simulink model "TosAeb"  (R2021a)
settings: Solver=FixedStepDiscrete  StartTime=0.0  StopTime=inf  FixedStep=0.01  RelTol=1e-3
undefined symbols (not defined in the file or in its folder): ACCModSts, AccSts1, AEB_ObjectMovingOrientation, AEB_ObjectMovingState, CN_BTCActive, CN_CurrentGearR, CN_CurrentGearR_DHT, CN_PTCActive, CN_VDCActive, CN_VSC_EspOff, CN_VSC_FCW_AEB_Error, CN_VSC_FCW_AEB_NoDispay, CN_VSC_FCW_AEB_PerfmDgdtn, CN_VSC_FCW_AEB_TempUnAvlbl, CN_VSC_VehStandstill, CNaccelAvoidMax, CNaccelAvoidMin, CNbrakePedalPress, CNcomfortStrLeftAccelMax, CNcomfortStrLeftAccelMin, CNcomfortStrRightAccelMax, CNcomfortStrRightAccelMin, CNDrvDoorOpen, CNDrvModeEco, CNDrvModeNorm, CNDrvModeOther, CNDrvModeSport, CNDrvSBRreminder, CNNull, CNobjInvalidID, CNPassDoorOpen, CNPassSBRreminder, CNPEBSAccelNegInf, CNPEBSAccelNull, CNPEBSTimeInf, CNPEBSTimeNull, CNSTOPMAX, CNSTOPNULL, CNsubjWidth, CNSwtOFF, CNSwtON, CNTTCMAX, CNTTCNULL, CNVehConfigAEBCycNone, CNVehConfigAEBNone, CNVehConfigAEBPedNone, CNVehConfigFCWNone, CNVehConfigJANone, CollisionSeverity, FALSE, FCWSnvty, JACycObjCalcInfoDefaultParams, JAPedObjCalcInfoDefaultParams, JAVehObjCalcInfoDefaultParams, latCycObjCalcInfoDefaultParams, latPedObjCalcInfoDefaultParams, longObjCalcInfoDefaultParams, ModSts, movingStatus, ObjectType ... (+1112)
libraries: simulink x3031, AEBSFunctionLib x50, Ta_Library x33, AEBSStateMachineLib x23, Mylibrary x12, simulink_extras x7, ObjSelect_lib x3, dspstat3 x2
(part 36 of 102)

== system TosAeb/AEB_Objsel/TA_InfoRebuild/TAObjInfoCalc/For Each Subsystem/Info_rebuild/Road_relative/LineInfo_Process/LineInfo_Process_Right ==
  Rightline_Data : Inport
  Bus Creator : BusCreator  Inputs=7
  Bus Selector : BusSelector  OutputSignals=lineSegNum,lineSegs
  Bus Selector1 : BusSelector  OutputSignals=lineSegValid,lineSegStart,lineSegEnd,lineC0,lineC1,lineC2,lineC3
  Bus Selector2 : BusSelector  OutputSignals=lineSegValid,lineSegStart,lineSegEnd,lineC0,lineC1,lineC2,lineC3
  Bus Selector3 : BusSelector  OutputSignals=lineSegValid,lineSegStart,lineSegEnd,lineC0,lineC1,lineC2,lineC3
  Data Type Conversion : DataTypeConversion  OutDataTypeStr=boolean
  Data Type Conversion1 : DataTypeConversion  OutDataTypeStr=boolean
  Data Type Conversion2 : DataTypeConversion  OutDataTypeStr=boolean
  Line_Info : MATLAB Function  TreatAsAtomicUnit=on
  Selector18 : Selector  IndexMode=Zero-based  InputPortWidth=3  IndexOptions=Index vector (dialog)  Indices=[0]  OutputSizes=1
  Selector2 : Selector  IndexMode=Zero-based  InputPortWidth=3  IndexOptions=Index vector (dialog)  Indices=[1]  OutputSizes=1
  Selector3 : Selector  IndexMode=Zero-based  InputPortWidth=3  IndexOptions=Index vector (dialog)  Indices=[2]  OutputSizes=1
  RightLine_Info : Outport
  wires:
  Rightline_Data/1 -> Bus Selector/1
  Selector3/1 -> Bus Selector3/1
  Selector18/1 -> Bus Selector1/1
  Selector2/1 -> Bus Selector2/1
  Bus Selector/2 -> Selector3/1, Selector2/1, Selector18/1  "<lineSegs>"
  Bus Selector1/1 -> Data Type Conversion/1  "<lineSegValid>"
  Bus Selector1/2 -> Line_Info/3(seg1_Start)  "<lineSegStart>"
  Bus Selector1/3 -> Line_Info/4(seg1_end)  "<lineSegEnd>"
  Bus Selector1/4 -> Line_Info/5(seg1_C0)  "<lineC0>"
  Bus Selector1/5 -> Line_Info/6(seg1_C1)  "<lineC1>"
  Bus Selector1/6 -> Line_Info/7(seg1_C2)  "<lineC2>"
  Bus Selector1/7 -> Line_Info/8(seg1_C3)  "<lineC3>"
  Bus Selector2/1 -> Data Type Conversion1/1  "<lineSegValid>"
  Bus Selector2/2 -> Line_Info/10(seg2_Start)  "<lineSegStart>"
  Bus Selector2/3 -> Line_Info/11(seg2_end)  "<lineSegEnd>"
  Bus Selector2/4 -> Line_Info/12(seg2_C0)  "<lineC0>"
  Bus Selector2/5 -> Line_Info/13(seg2_C1)  "<lineC1>"
  Bus Selector2/6 -> Line_Info/14(seg2_C2)  "<lineC2>"
  Bus Selector2/7 -> Line_Info/15(seg2_C3)  "<lineC3>"
  Bus Selector3/1 -> Data Type Conversion2/1  "<lineSegValid>"
  Bus Selector3/2 -> Line_Info/17(seg3_Start)  "<lineSegStart>"
  Bus Selector3/3 -> Line_Info/18(seg3_end)  "<lineSegEnd>"
  Bus Selector3/4 -> Line_Info/19(seg3_C0)  "<lineC0>"
  Bus Selector3/5 -> Line_Info/20(seg3_C1)  "<lineC1>"
  Bus Selector3/6 -> Line_Info/21(seg3_C2)  "<lineC2>"
  Bus Selector3/7 -> Line_Info/22(seg3_C3)  "<lineC3>"
  Bus Selector/1 -> Line_Info/1(seg_num)  "<lineSegNum>"
  Line_Info/1(Valid) -> Bus Creator/1
  Line_Info/2(Line_start) -> Bus Creator/2
  Line_Info/3(Line_end) -> Bus Creator/3
  Line_Info/4(C0) -> Bus Creator/4
  Line_Info/5(C1) -> Bus Creator/5
  Line_Info/6(C2) -> Bus Creator/6
  Line_Info/7(C3) -> Bus Creator/7
  Bus Creator/1 -> RightLine_Info/1
  Data Type Conversion/1 -> Line_Info/2(seg1_valid)
  Data Type Conversion1/1 -> Line_Info/9(seg2_valid)
  Data Type Conversion2/1 -> Line_Info/16(seg3_valid)

== system TosAeb/AEB_Objsel/TA_InfoRebuild/TAObjInfoCalc/For Each Subsystem/Info_rebuild/Road_relative/RebuildInfo_Process/If Action Subsystem ==
  Action Port : ActionPort  ActionPortLabel=if(u1 > 0)
  Constant : Constant  Value=0  OutDataTypeStr=single
  Constant1 : Constant  Value=0  OutDataTypeStr=single
  Constant2 : Constant  Value=0  OutDataTypeStr=uint8
  objLatDist2LaneCenter : Outport
  objLatDist2EgoCenter : Outport  Port=2
  objPosition : Outport  Port=3
  wires:
  Constant2/1 -> objPosition/1
  Constant1/1 -> objLatDist2EgoCenter/1
  Constant/1 -> objLatDist2LaneCenter/1

== system TosAeb/AEB_Objsel/TA_InfoRebuild/TAObjInfoCalc/For Each Subsystem/Info_rebuild/Road_relative/RebuildInfo_Process/If Action Subsystem1 ==
  Obj_Infomation : Inport
  LeftLine_Info : Inport  Port=2
  RightLine_Info : Inport  Port=3
  Action Port : ActionPort  ActionPortLabel=else
  Bus Selector : BusSelector  OutputSignals=PosX_s,PosY_s
  Bus Selector1 : BusSelector  OutputSignals=Valid,C0,C1,C2,C3
  Bus Selector2 : BusSelector  OutputSignals=Valid,C0,C1,C2,C3
  MATLAB Function : MATLAB Function  TreatAsAtomicUnit=on
  objLatDist2LaneCenter : Outport
  objLatDist2EgoCenter : Outport  Port=2
  objPosition : Outport  Port=3
  wires:
  Obj_Infomation/1 -> Bus Selector/1
  LeftLine_Info/1 -> Bus Selector1/1
  RightLine_Info/1 -> Bus Selector2/1
  Bus Selector/1 -> MATLAB Function/1(dx)  "<PosX_s>"
  Bus Selector/2 -> MATLAB Function/2(dy)  "<PosY_s>"
  Bus Selector1/2 -> MATLAB Function/4(left_C0)  "<C0>"
  Bus Selector1/1 -> MATLAB Function/3(left_valid)  "<Valid>"
  Bus Selector1/3 -> MATLAB Function/5(left_C1)  "<C1>"
  Bus Selector1/4 -> MATLAB Function/6(left_C2)  "<C2>"
  Bus Selector1/5 -> MATLAB Function/7(left_C3)  "<C3>"
  Bus Selector2/1 -> MATLAB Function/8(right_valid)  "<Valid>"
  Bus Selector2/2 -> MATLAB Function/9(right_C0)  "<C0>"
  Bus Selector2/3 -> MATLAB Function/10(right_C1)  "<C1>"
  Bus Selector2/4 -> MATLAB Function/11(right_C2)  "<C2>"
  Bus Selector2/5 -> MATLAB Function/12(right_C3)  "<C3>"
  MATLAB Function/1(objLatDist2LaneCenter) -> objLatDist2LaneCenter/1
  MATLAB Function/2(objLatDist2EgoCenter) -> objLatDist2EgoCenter/1
  MATLAB Function/3(objPosition) -> objPosition/1

== system TosAeb/AEB_Objsel/TA_InfoRebuild/TAObjInfoCalc/For Each Subsystem/Info_rebuild/Road_relative/RebuildInfo_Process/Subsystem ==
  Obj_Infomation : Inport
  Bus Selector : BusSelector  OutputSignals=ID,Valid
  Compare To Constant : simulink/Logic and Bit Operations/Compare To Constant  relop===  const=0  OutDataTypeStr=boolean
  Compare To Constant1 : simulink/Logic and Bit Operations/Compare To Constant  relop===  const=0  OutDataTypeStr=boolean
  Data Type Conversion : DataTypeConversion  OutDataTypeStr=uint8
  Logical Operator : Logic  Operator=OR  AllPortsSameDT=off  OutDataTypeStr=boolean
  if_judge : Outport
  wires:
  Obj_Infomation/1 -> Bus Selector/1
  Bus Selector/1 -> Compare To Constant/1  "<ID>"
  Bus Selector/2 -> Compare To Constant1/1  "<Valid>"
  Compare To Constant/1 -> Logical Operator/1
  Compare To Constant1/1 -> Logical Operator/2
  Logical Operator/1 -> Data Type Conversion/1
  Data Type Conversion/1 -> if_judge/1

== system TosAeb/AEB_Objsel/TA_Processor/AEB_ObjSelect/TargetInfoCheck/Data_precess/TA_EffectCalculate/Obj_FilterFlgCalculate/JA_ObjFilter ==
  TA_ObjInfo : Inport
  PahEstimn : Inport  Port=2
  VehSelfData : Inport  Port=3
  Bus Selector : BusSelector  OutputSignals=VehSelf.ABS_VehicleSpeed,VehSelf.AX1,VehSelf.AY1,VehSelf.YawRate
  Bus Selector1 : BusSelector  OutputSignals=TA_ObsCenterAngle,TA_ObsOrientation,TA_ObsLength,TA_ObsWidth,TA_ObsDx,TA_ObsDy,TA_ObsAy,TA_ObsID,TA_ObsVy,TA_ObsVxAbs,TA_ObsAxAbs
  CollisionProbCalc : Subsystem
  CollisionProbCalcFlow : Stateflow chart  TreatAsAtomicUnit=on
  Constant1 : Constant  Value=100  OutDataTypeStr=single
  Constant2 : Constant  Value=0.02  OutDataTypeStr=single
  Constant3 : Constant  Value=2  OutDataTypeStr=single
  JAObjEnable : Subsystem
  Logical Operator : Logic  Operator=NOT  AllPortsSameDT=off  OutDataTypeStr=boolean
  Switch : Switch  Criteria=u2 ~= 0
  ObjColliInfo : Outport
  wires:
  VehSelfData/1 -> Bus Selector/1
  Bus Selector/2 -> CollisionProbCalc/3(subjLongAccel)  "<AX1>"
  [CrossFlg] -> CollisionProbCalc/Trigger
  PahEstimn/1 -> CollisionProbCalc/17(PahEstimn)
  CollisionProbCalc/1(ObjColliFlg) -> CollisionProbCalcFlow/3(preCollisionProb)  "ObjColliFlg"
  CollisionProbCalcFlow/1(TTC) -> Switch/3  "tPredictTime"
  Bus Selector1/2 -> CollisionProbCalc/7(ObjectMovingOrientation)  "<TA_ObsOrientation>"
  Constant3/1 -> CollisionProbCalcFlow/2(P_JaPedCA_PreCollisionPredTimeMax)
  CollisionProbCalcFlow/3(CollisionProbFunc()) -> [CrossFlg]
  Bus Selector/3 -> CollisionProbCalc/4(subjLatAccel)  "<AY1>"
  Bus Selector/4 -> CollisionProbCalc/5(subjYawRate)  "<YawRate>"
  CollisionProbCalcFlow/2(tPredictTimeOut) -> CollisionProbCalc/1(tPredTime)
  Bus Selector1/1 -> CollisionProbCalc/6(ObjectYawAngle)  "<TA_ObsCenterAngle>"
  Constant2/1 -> CollisionProbCalcFlow/1(P_JaPedCA_PreCollisionStepTime)
  Bus Selector1/3 -> CollisionProbCalc/8(ObjectLength)  "<TA_ObsLength>"
  Bus Selector/1 -> CollisionProbCalc/2(subjVel)  "<ABS_VehicleSpeed>"
  Bus Selector1/4 -> CollisionProbCalc/9(ObjectWidth)  "<TA_ObsWidth>"
  Bus Selector1/5 -> CollisionProbCalc/10(dx)  "<TA_ObsDx>"
  Bus Selector1/6 -> CollisionProbCalc/11(dy)  "<TA_ObsDy>"
  Bus Selector1/7 -> CollisionProbCalc/12(ay)  "<TA_ObsAy>"
  Bus Selector1/8 -> CollisionProbCalc/13(ObjectID)  "<TA_ObsID>"
  Bus Selector1/9 -> CollisionProbCalc/14(vy)  "<TA_ObsVy>"
  Bus Selector1/10 -> CollisionProbCalc/15(vxAbsObj)  "<TA_ObsVxAbs>"
  Bus Selector1/11 -> CollisionProbCalc/16(axAbsObj)  "<TA_ObsAxAbs>"
  TA_ObjInfo/1 -> JAObjEnable/1(TA_ObjInfo), [TA_ObjInfo]
  [TA_ObjInfo] -> Bus Selector1/1
  JAObjEnable/1(JAObjEnable) -> Logical Operator/1
  Logical Operator/1 -> Switch/2
  Switch/1 -> ObjColliInfo/1
  Constant1/1 -> Switch/1  "ObjColliFlg"

== system TosAeb/AEB_Objsel/TA_Processor/AEB_ObjSelect/TargetInfoCheck/Data_precess/TA_EffectCalculate/Obj_FilterFlgCalculate/Lat_ObjFilter ==
  Utility : Inport
  TA_ObjInfo : Inport  Port=2
  VehSelfData : Inport  Port=3
  If : If
  Lat_Objelse : Subsystem  TreatAsAtomicUnit=on
  Lat_Objif : Subsystem  TreatAsAtomicUnit=on
  Merge : Merge
  Merge1 : Merge
  Merge2 : Merge
  Subsystem : Subsystem
  LatObj_Valid : Outport
  Lat_ObjIntersect : Outport  Port=2
  Lat_TTR : Outport  Port=3
  wires:
  Subsystem/1(Obj_IDFlg) -> If/1
  Utility/1 -> Subsystem/1(TA_ObjID)
  If/1 -> Lat_Objif/Action
  If/2 -> Lat_Objelse/Action
  VehSelfData/1 -> Lat_Objelse/2(VehSelfData)
  TA_ObjInfo/1 -> Lat_Objelse/1(TA_ObjInfo)
  Lat_Objif/1(LatObj_Valid) -> Merge/1
  Lat_Objelse/1(LatObj_Valid) -> Merge/2
  Merge/1 -> LatObj_Valid/1
  Lat_Objif/2(Lat_ObjIntersect) -> Merge1/1
  Lat_Objelse/2(Lat_ObjIntersect) -> Merge1/2
  Lat_Objif/3(Lat_TTR) -> Merge2/1
  Lat_Objelse/3(Lat_TTR) -> Merge2/2
  Merge1/1 -> Lat_ObjIntersect/1
  Merge2/1 -> Lat_TTR/1

== system TosAeb/AEB_Objsel/TA_Processor/AEB_ObjSelect/TargetInfoCheck/Data_precess/TA_EffectCalculate/Obj_FilterFlgCalculate/Long_ObjFilter ==
  Obj_WeightCoef : Inport
  TA_ObjInfo : Inport  Port=2
  VehSelfData : Inport  Port=3
  " PahEstimn" : Inport  Port=4
  Info_Calculate : Subsystem
  OverlapCalculate : Subsystem
  TTR_Calculate : Subsystem
  Boudingbox_Calculate : Outport
  Obj_Coef : Outport  Port=2
  TTI : Outport  Port=3
  Long_ObjWeightCoef : Outport  Port=4
  wires:
  Obj_WeightCoef/1 -> Info_Calculate/1(Obj_WeightCoef)
  TA_ObjInfo/1 -> Info_Calculate/2(TA_ObjInfo), [TA_ObjInfo]
  VehSelfData/1 -> [VehSelfData], Info_Calculate/3(VehSelfData)
  Info_Calculate/1(Long_ObjWeightCoef) -> Long_ObjWeightCoef/1, TTR_Calculate/1(Long_ObjWeightCoef)
  TTR_Calculate/2(TimeToReachLgt) -> [TTI], OverlapCalculate/2(TimeToReachLgt)
  TTR_Calculate/1(Long_ObjWeightCoefTTI) -> OverlapCalculate/1(Long_ObjWeightCoefTTI), [Obj_Coef]
  [TA_ObjInfo] -> TTR_Calculate/2(TA_ObjInfo)
  [TA_ObjInfo] -> OverlapCalculate/4(TA_ObjInfo)
  [VehSelfData] -> TTR_Calculate/3(VehSelfData)
  [VehSelfData] -> OverlapCalculate/5(VehSelfData)
  " PahEstimn"/1 -> OverlapCalculate/3(PahEstimn)
  OverlapCalculate/1(Boudingbox_Calculate) -> Boudingbox_Calculate/1
  [Obj_Coef] -> Obj_Coef/1
  [TTI] -> TTI/1

== system TosAeb/AEB_Objsel/TA_Processor/AEB_ObjSelect/TargetInfoCheck/Data_precess/TA_EffectCalculate/Obj_FilterFlgCalculate/WeightCoef_Cal ==
  note: 是否要根据有效目标降低循环维度，以减少for循环运算次数
  Overlap_Current : Inport
  Utility : Inport  Port=2
  Overlap_Prediction : Inport  Port=3
  VehSelfData : Inport  Port=4
  Bus Selector : BusSelector  OutputSignals=VehSelf.AEB_Intervention_ESP
  Compare To Constant : simulink/Logic and Bit Operations/Compare To Constant  relop===  const=0  OutDataTypeStr=boolean
  Compare To Constant{1,4..7} (5 alike) : simulink/Logic and Bit Operations/Compare To Constant  relop===  const=1  OutDataTypeStr=boolean
  Compare To Constant2 : simulink/Logic and Bit Operations/Compare To Constant  relop===  const=0  OutDataTypeStr=boolean
  Compare To Constant3 : simulink/Logic and Bit Operations/Compare To Constant  relop===  const=0  OutDataTypeStr=boolean
  Constant : Constant  Value=255  OutDataTypeStr=uint8
  Constant1 : Constant  OutDataTypeStr=uint8
  Constant2 : Constant  Value=2  OutDataTypeStr=uint8
  Constant3 : Constant  Value=3  OutDataTypeStr=uint8
  Constant4 : Constant  Value=0  OutDataTypeStr=uint8
  Logical Operator : Logic  AllPortsSameDT=off  OutDataTypeStr=boolean
  Logical Operator1 : Logic  AllPortsSameDT=off  OutDataTypeStr=boolean
  Logical Operator2 : Logic  AllPortsSameDT=off  OutDataTypeStr=boolean
  Switch{,1..4} (5 alike) : Switch  Criteria=u2 ~= 0
  WeightCoef_Cal1 : MATLAB Function  Commented=on  TreatAsAtomicUnit=on
  Obj_WeightCoef : Outport
  wires:
  Utility/1 -> Compare To Constant/1
  Compare To Constant/1 -> Switch/2
  Constant/1 -> Switch/1
  Overlap_Current/1 -> Compare To Constant5/1, Compare To Constant3/1, Compare To Constant1/1
  Overlap_Prediction/1 -> Compare To Constant6/1, Compare To Constant4/1, Compare To Constant2/1
  Compare To Constant1/1 -> Switch1/1, Logical Operator/1
  Compare To Constant2/1 -> Logical Operator/2
  Logical Operator/1 -> Switch2/2
  Constant1/1 -> Switch2/1
  Logical Operator1/1 -> Switch3/2
  Compare To Constant3/1 -> Logical Operator1/1
  Compare To Constant4/1 -> Logical Operator1/2
  Constant2/1 -> Switch3/1
  Compare To Constant5/1 -> Logical Operator2/1
  Compare To Constant6/1 -> Logical Operator2/2
  Logical Operator2/1 -> Switch4/2
  Constant3/1 -> Switch4/1
  Switch4/1 -> Switch3/3
  Switch3/1 -> Switch2/3
  Switch2/1 -> Switch1/3
  Constant4/1 -> Switch4/3
  Switch/1 -> Obj_WeightCoef/1
  VehSelfData/1 -> Bus Selector/1
  Compare To Constant7/1 -> Switch1/2
  Switch1/1 -> Switch/3
  Bus Selector/1 -> Compare To Constant7/1  "<AEB_Intervention_ESP>"

== system TosAeb/AEB_Objsel/TA_Processor/AEB_ObjSelect/TargetInfoCheck/Data_precess/TA_EffectCalculate/ValideateObj/ObjPos_Calculate ==
  TA_ObsInfo : Inport
  Obj_Enable : Inport  Port=2
  PathEstimn : Inport  Port=3
  If : If
  Merge : Merge
  Merge1 : Merge
  ObjPos_Calculateelse : Subsystem  TreatAsAtomicUnit=on
  ObjPos_Calculateif : Subsystem  TreatAsAtomicUnit=on
  Subsystem : Subsystem
  Utility : Outport
  Overlap_Current : Outport  Port=2
  Overlap_Prediction : Outport  Port=3
  wires:
  Obj_Enable/1 -> Subsystem/1(Obj_Enable)
  Subsystem/2(Obj_Flg) -> If/1
  If/1 -> ObjPos_Calculateif/Action
  If/2 -> ObjPos_Calculateelse/Action
  TA_ObsInfo/1 -> ObjPos_Calculateelse/1(TA_ObsInfo)
  PathEstimn/1 -> ObjPos_Calculateelse/2(PathEstimn)
  Subsystem/1(Utility) -> Utility/1
  ObjPos_Calculateif/1(Overlap_Current) -> Merge/1
  ObjPos_Calculateelse/1(Overlap_Current) -> Merge/2
  ObjPos_Calculateif/2(Overlap_Prediction) -> Merge1/1
  ObjPos_Calculateelse/2(Overlap_Prediction) -> Merge1/2
  Merge/1 -> Overlap_Current/1
  Merge1/1 -> Overlap_Prediction/1

== system TosAeb/AEB_Objsel/TA_Processor/AEB_ObjSelect/TargetInfoCheck/Data_precess/TA_EffectCalculate/ValideateObj/ValidObj_PreProcess ==
  TA_ObjInfo : Inport
  VehSelfData : Inport  Port=2
  Bus Selector : BusSelector  OutputSignals=TA_ObsType,TA_ObsOrientation,TA_ObsDx,TA_ObsDy,TA_ObsVx,TA_ObsConfidence,TA_ObsVxAbs
  Bus Selector1 : BusSelector  OutputSignals=TA_ObsID
  Bus Selector2 : BusSelector  OutputSignals=VehSelf.ABS_VehicleSpeed
  If : If
  If Action Subsystem : Subsystem  TreatAsAtomicUnit=on
  If Action Subsystem1 : Subsystem  TreatAsAtomicUnit=on
  Merge : Merge
  Subsystem : Subsystem
  Obj_Enable : Outport
  wires:
  Subsystem/1(Obj_IDFlg) -> If/1
  If/1 -> If Action Subsystem/Action
  If/2 -> If Action Subsystem1/Action
  If Action Subsystem/1(Obj_Enable) -> Merge/1
  If Action Subsystem1/1(Obj_Enable) -> Merge/2
  Merge/1 -> Obj_Enable/1
  TA_ObjInfo/1 -> Bus Selector/1, Bus Selector1/1
  VehSelfData/1 -> Bus Selector2/1
  Bus Selector2/1 -> If Action Subsystem1/8(Veh_LongSpd_mps)  "<ABS_VehicleSpeed>"
  Bus Selector1/1 -> Subsystem/1(TA_ObsID)  "<TA_ObsID>"
  Bus Selector/1 -> If Action Subsystem1/1(TA_ObsType)  "<TA_ObsType>"
  Bus Selector/2 -> If Action Subsystem1/2(TA_ObjBehavior)  "<TA_ObsOrientation>"
  Bus Selector/3 -> If Action Subsystem1/3(TA_Obsdx)  "<TA_ObsDx>"
  Bus Selector/4 -> If Action Subsystem1/4(TA_Obsdy)  "<TA_ObsDy>"
  Bus Selector/5 -> If Action Subsystem1/5(TA_Obsvx)  "<TA_ObsVx>"
  Bus Selector/6 -> If Action Subsystem1/6(TA_ObsQuality)  "<TA_ObsConfidence>"
  Bus Selector/7 -> If Action Subsystem1/7(TA_ObsVxAbs)  "<TA_ObsVxAbs>"

== system TosAeb/AEB_Objsel/TA_Processor/PreProcessor/ObjectPrecessor/TAObjInfoCalc/For Each Subsystem/ForEachInput/PosErrorCalc ==
  Pos_x : Inport
  ObsID_u8 : Inport  Port=2
  Add : Sum
  Add1 : Sum  Inputs=+-
  Compare To Constant3 : simulink/Logic and Bit Operations/Compare To Constant  relop=<  const=0  OutDataTypeStr=boolean
  Constant : Constant  Value=262  OutDataTypeStr=single
  Constant1 : Constant  Value=P_TA_PrmForVehRearAxleDisttoFrnt  OutDataTypeStr=single
  FakeObjJudge : Stateflow chart  TreatAsAtomicUnit=on
  Logical Operator : Logic  Operator=NOT  AllPortsSameDT=off  OutDataTypeStr=boolean
  Max : MinMax  Function=max  Inputs=2
  Switch : Switch  Criteria=u2 ~= 0
  Unary Minus : UnaryMinus
  Unit Delay2 : UnitDelay  SampleTime=-1  HasFrameUpgradeWarning=on
  ObsPosX_s : Outport
  wires:
  ObsID_u8/1 -> Unit Delay2/1, FakeObjJudge/1(ID)
  Unit Delay2/1 -> FakeObjJudge/2(LastID)
  Pos_x/1 -> [Pos_x], Switch/3, Compare To Constant3/1
  Compare To Constant3/1 -> FakeObjJudge/3(FakeFlg)
  FakeObjJudge/1(ConfFlg) -> Logical Operator/1
  Logical Operator/1 -> Switch/2
  [Pos_x] -> Unary Minus/1
  Unary Minus/1 -> Add/1
  Add/1 -> Switch/1
  Constant/1 -> Add/2
  Constant1/1 -> Add1/2
  Switch/1 -> Max/1
  Max/1 -> Add1/1
  [Pos_x] -> Max/2
  Add1/1 -> ObsPosX_s/1

== system TosAeb/AEB_Objsel/TA_Processor/PreProcessor/ObjectPrecessor/TAObjInfoCalc/For Each Subsystem/Info_Check1/BasicInfo_check ==
  ID_change : Inport
  Fus_ObjState : Inport  Port=2
  Fus_Type : Inport  Port=3
  ObsID_u8 : Inport  Port=4
  ObsConfidence_u8 : Inport  Port=5
  ObsPosX_s : Inport  Port=6
  ObsPosY_s : Inport  Port=7
  ObsVelX_s : Inport  Port=8
  ObsVelY_s : Inport  Port=9
  ObsAccX_s : Inport  Port=10
  ObsPosXSTD_s : Inport  Port=11
  ObsPosYSTD_s : Inport  Port=12
  ObsVelXSTD_s : Inport  Port=13
  ObsVelYSTD_s : Inport  Port=14
  ObsAccY_s : Inport  Port=15
  ObsVelXAbs_s : Inport  Port=16
  ObsVelYAbs_s : Inport  Port=17
  ObsAngle_s : Inport  Port=18
  ObsAngleSTD_s : Inport  Port=19
  Veh_AEBState : Inport  Port=20
  Vhe_Spd : Inport  Port=21
  Veh_YawRate : Inport  Port=22
  Veh_DrvAcc : Inport  Port=23
  Veh_DrvAy : Inport  Port=24
  AbsSpd : Subsystem
  AccCorrect : Subsystem
  DxCorrect : Subsystem
  DyCorrect : Subsystem
  HeadAngle : Subsystem
  IDProcess : Subsystem
  QuaCorrect : Subsystem
  VxCorrect : Subsystem
  VyCorrect : Subsystem
  Fus_ObjDy : Outport
  Fus_ObjDx : Outport  Port=2
  Fus_ObjVx : Outport  Port=3
  Fus_ObjVy : Outport  Port=4
  Fus_ObjQuality : Outport  Port=5
  Fus_ObjAy : Outport  Port=6
  Fus_ObjAx : Outport  Port=7
  Fus_ObjVxAbs : Outport  Port=8
  Fus_ObjVyAbs : Outport  Port=9
  Fus_ObjHeadAngle : Outport  Port=10
  wires:
  [Obj_VxCr] -> AccCorrect/6(Obj_Vx)
  [Obj_Vy] -> DyCorrect/5(Fus_ObjVy_Single)
  ObsVelXSTD_s/1 -> VxCorrect/3(Fus_ObjVxStd)
  DxCorrect/2(Dx_CorrectFlg) -> [dx_Flg]
  ObsPosYSTD_s/1 -> DyCorrect/3(Fus_ObsDyStd)
  [Obj_ID] -> DxCorrect/1(Obj_ID)
  [dy_Flg] -> QuaCorrect/4(dy_flg)
  [Veh_AEBSta] -> DxCorrect/5(Veh_AEBFunState)
  DxCorrect/1(Fus_ObjDx) -> Fus_ObjDx/1
  [Obj_Dx] -> VxCorrect/4(Fus_ObjDx_Single)
  [Veh_AEBSta] -> VyCorrect/7(Veh_AEBFunState)
  ObsVelYSTD_s/1 -> VyCorrect/5(Fus_ObsVyStd)
  [Obj_Vx] -> DxCorrect/4(Fus_ObjVx_Single)
  ObsConfidence_u8/1 -> QuaCorrect/2(ObsConfidence_u8)
  VxCorrect/1(Fus_ObjVx) -> Fus_ObjVx/1, [Obj_VxCr]
  VxCorrect/2(Vx_CorrectFlg) -> [vx_Flg]
  [vy_Flg] -> QuaCorrect/7(vy_flg)
  ObsPosXSTD_s/1 -> DxCorrect/3(Fus_ObjDxQua_Single)
  [dx_Flg] -> QuaCorrect/5(dx_flg)
  [vx_Flg] -> QuaCorrect/6(vx_flg)
  QuaCorrect/1(Fus_ObjQuality) -> Fus_ObjQuality/1
  DyCorrect/2(Dy_CorrectFlg) -> [dy_Flg]
  VyCorrect/2(Vy_CorrectFlg) -> [vy_Flg]
  DyCorrect/1(Fus_ObjDy) -> Fus_ObjDy/1
  [Obj_ID] -> QuaCorrect/1(Obj_ID)
  AccCorrect/1(Fus_ObjAy) -> Fus_ObjAy/1
  Veh_AEBState/1 -> [Veh_AEBSta], DyCorrect/4(Veh_AEBFunState)
  [Veh_AEBSta] -> VxCorrect/5(Veh_AEBFunState)
  [Obj_ID] -> VyCorrect/1(Obj_ID)
  AccCorrect/2(Fus_ObjAx) -> Fus_ObjAx/1
  [Obj_ID] -> VxCorrect/1(Obj_ID)
  ObsPosX_s/1 -> [Obj_Dx], DxCorrect/2(Fus_ObjDx_Single)
  ObsVelX_s/1 -> VxCorrect/2(Fus_ObjVx_Single), [Obj_Vx]
  Fus_ObjState/1 -> VyCorrect/3(Fus_TargetState)
  ObsVelY_s/1 -> [Obj_Vy], VyCorrect/2(Fus_ObjVy_Single)
  [Obj_VyCr] -> AccCorrect/5(Obj_vy)
  VyCorrect/1(Fus_ObjVy) -> [Obj_VyCr], Fus_ObjVy/1
  [Veh_AEBSta] -> AccCorrect/7(Veh_AEBSta)
  ObsPosY_s/1 -> DyCorrect/2(Fus_ObjDy_Single), [Obj_Dy]
  [Obj_Dy] -> VyCorrect/6(Fus_ObjDy_Single)
  ID_change/1 -> IDProcess/1(ID_change)
  ObsID_u8/1 -> IDProcess/2(ObsID_u8)
  IDProcess/1(Obs_ID) -> [Obj_ID], DyCorrect/1(Obj_ID)
  Veh_YawRate/1 -> [Veh_YawRate], VyCorrect/4(Veh_YawRate)
  ObsAccX_s/1 -> AccCorrect/1(ObsAccX_s)
  ObsAccY_s/1 -> AccCorrect/2(ObsAccY_s)
  Veh_DrvAcc/1 -> AccCorrect/3(Veh_AccX)
  Veh_DrvAy/1 -> AccCorrect/4(Veh_AccY)
  [Obj_VyCr] -> AbsSpd/6(Obj_VyCr)
  [Obj_VxCr] -> AbsSpd/5(Obj_VxCr)
  [Veh_YawRate] -> AbsSpd/4(Veh_YawRate)
  Vhe_Spd/1 -> AbsSpd/3(Vhe_Spd)
  ObsVelXAbs_s/1 -> AbsSpd/1(ObsVelXAbs_s)
  ObsVelYAbs_s/1 -> AbsSpd/2(ObsVelYAbs_s)
  [Veh_AEBSta] -> QuaCorrect/3(Veh_AEBFunState)
  AbsSpd/1(Fus_ObjVxAbs) -> Fus_ObjVxAbs/1
  AbsSpd/2(Fus_ObjVyAbs) -> Fus_ObjVyAbs/1
  Fus_Type/1 -> HeadAngle/2(Fus_Type)
  ObsAngle_s/1 -> HeadAngle/3(ObsAngle_s)
  ObsAngleSTD_s/1 -> HeadAngle/4(ObsAngleSTD_s)
  [Obj_VxCr] -> HeadAngle/5(Obj_VxCr)
  [Obj_VyCr] -> HeadAngle/6(Obj_VyCr)
  [Obj_ID] -> HeadAngle/1(Obj_ID)
  HeadAngle/1(Fus_ObjHeadAngle) -> Fus_ObjHeadAngle/1

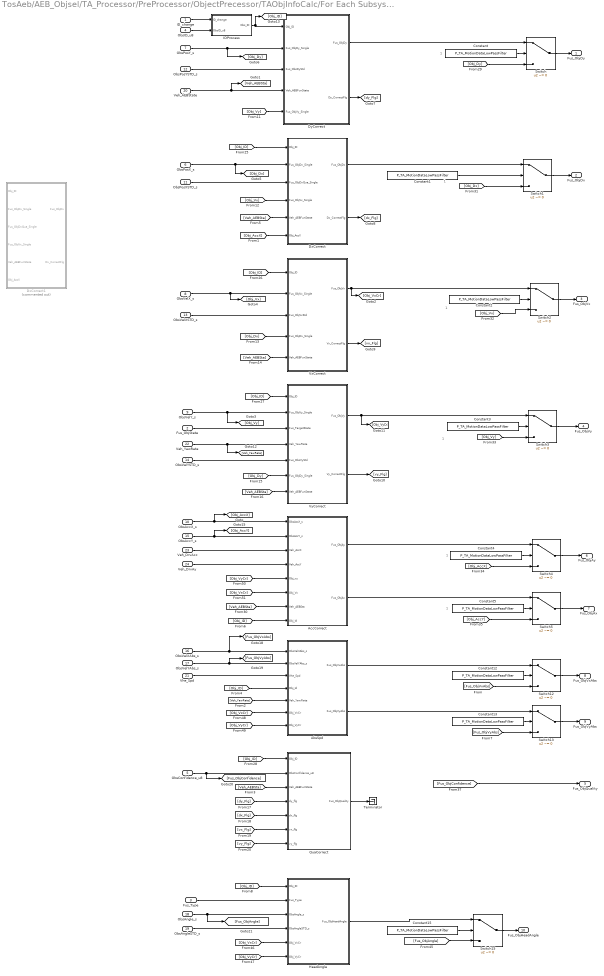
== system TosAeb/AEB_Objsel/TA_Processor/PreProcessor/ObjectPrecessor/TAObjInfoCalc/For Each Subsystem/Info_Check1/Basic_Info_check ==
  note: 1
  note: 1
  note: 1
  note: 1
  note: 1
  note: 1
  ID_change : Inport
  Fus_ObjState : Inport  Port=2
  Fus_Type : Inport  Port=3
  ObsID_u8 : Inport  Port=4
  ObsConfidence_u8 : Inport  Port=5
  ObsPosX_s : Inport  Port=6
  ObsPosY_s : Inport  Port=7
  ObsVelX_s : Inport  Port=8
  ObsVelY_s : Inport  Port=9
  ObsAccX_s : Inport  Port=10
  ObsPosXSTD_s : Inport  Port=11
  ObsPosYSTD_s : Inport  Port=12
  ObsVelXSTD_s : Inport  Port=13
  ObsVelYSTD_s : Inport  Port=14
  ObsAccY_s : Inport  Port=15
  ObsVelXAbs_s : Inport  Port=16
  ObsVelYAbs_s : Inport  Port=17
  ObsAngle_s : Inport  Port=18
  ObsAngleSTD_s : Inport  Port=19
  Veh_AEBState : Inport  Port=20
  Vhe_Spd : Inport  Port=21
  Veh_YawRate : Inport  Port=22
  Veh_DrvAcc : Inport  Port=23
  Veh_DrvAy : Inport  Port=24
  AbsSpd : Subsystem
  AccCorrect : Subsystem
  Constant{,1..5,12..13,15} (9 alike) : Constant  Value=P_TA_MotionDataLowPassFilter
  DxCorrect : Subsystem
  DxCorrect1 : Subsystem  Commented=on
  DyCorrect : Subsystem
  HeadAngle : Subsystem
  IDProcess : Subsystem
  QuaCorrect : Subsystem
  Switch{,1..5,12..13,15} (9 alike) : Switch  Criteria=u2 ~= 0
  Terminator : Terminator
  VxCorrect : Subsystem
  VyCorrect : Subsystem
  Fus_ObjDy : Outport
  Fus_ObjDx : Outport  Port=2
  Fus_ObjVx : Outport  Port=3
  Fus_ObjVy : Outport  Port=4
  Fus_ObjQuality : Outport  Port=5
  Fus_ObjAy : Outport  Port=6
  Fus_ObjAx : Outport  Port=7
  Fus_ObjVxAbs : Outport  Port=8
  Fus_ObjVyAbs : Outport  Port=9
  Fus_ObjHeadAngle : Outport  Port=10
  wires:
  [Obj_Vy] -> DyCorrect/5(Fus_ObjVy_Single)
  ObsVelXSTD_s/1 -> VxCorrect/3(Fus_ObjVxStd)
  DxCorrect/2(Dx_CorrectFlg) -> [dx_Flg]
  ObsPosYSTD_s/1 -> DyCorrect/3(Fus_ObsDyStd)
  [Obj_ID] -> DxCorrect/1(Obj_ID)
  [dy_Flg] -> QuaCorrect/4(dy_flg)
  [Veh_AEBSta] -> DxCorrect/5(Veh_AEBFunState)
  DxCorrect/1(Fus_ObjDx) -> Switch1/1
  [Obj_Dx] -> VxCorrect/4(Fus_ObjDx_Single)
  [Veh_AEBSta] -> VyCorrect/7(Veh_AEBFunState)
  ObsVelYSTD_s/1 -> VyCorrect/5(Fus_ObsVyStd)
  [Obj_Vx] -> DxCorrect/4(Fus_ObjVx_Single)
  ObsConfidence_u8/1 -> [Fus_ObjConfidence], QuaCorrect/2(ObsConfidence_u8)
  VxCorrect/1(Fus_ObjVx) -> [Obj_VxCr], Switch2/1
  VxCorrect/2(Vx_CorrectFlg) -> [vx_Flg]
  [vy_Flg] -> QuaCorrect/7(vy_flg)
  ObsPosXSTD_s/1 -> DxCorrect/3(Fus_ObjDxQua_Single)
  [dx_Flg] -> QuaCorrect/5(dx_flg)
  [vx_Flg] -> QuaCorrect/6(vx_flg)
  DyCorrect/2(Dy_CorrectFlg) -> [dy_Flg]
  VyCorrect/2(Vy_CorrectFlg) -> [vy_Flg]
  DyCorrect/1(Fus_ObjDy) -> Switch/1
  [Obj_ID] -> QuaCorrect/1(Obj_ID)
  Veh_AEBState/1 -> [Veh_AEBSta], DyCorrect/4(Veh_AEBFunState)
  [Veh_AEBSta] -> VxCorrect/5(Veh_AEBFunState)
  [Obj_ID] -> VyCorrect/1(Obj_ID)
  [Obj_ID] -> VxCorrect/1(Obj_ID)
  ObsPosX_s/1 -> [Obj_Dx], DxCorrect/2(Fus_ObjDx_Single)
  ObsVelX_s/1 -> [Obj_Vx], VxCorrect/2(Fus_ObjVx_Single)
  Fus_ObjState/1 -> VyCorrect/3(Fus_TargetState)
  ObsVelY_s/1 -> VyCorrect/2(Fus_ObjVy_Single), [Obj_Vy]
  VyCorrect/1(Fus_ObjVy) -> [Obj_VyCr], Switch3/1
  [Veh_AEBSta] -> AccCorrect/7(Veh_AEBSta)
  ObsPosY_s/1 -> [Obj_Dy], DyCorrect/2(Fus_ObjDy_Single)
  [Obj_Dy] -> VyCorrect/6(Fus_ObjDy_Single)
  ID_change/1 -> IDProcess/1(ID_change)
  ObsID_u8/1 -> IDProcess/2(ObsID_u8)
  IDProcess/1(Obs_ID) -> [Obj_ID], DyCorrect/1(Obj_ID)
  Veh_YawRate/1 -> VyCorrect/4(Veh_YawRate), [Veh_YawRate]
  ObsAccX_s/1 -> [Obj_AccX], AccCorrect/1(ObsAccX_s)
  ObsAccY_s/1 -> [Obj_AccY], AccCorrect/2(ObsAccY_s)
  Veh_DrvAcc/1 -> AccCorrect/3(Veh_AccX)
  Veh_DrvAy/1 -> AccCorrect/4(Veh_AccY)
  [Veh_YawRate] -> AbsSpd/5(Veh_YawRate)
  Vhe_Spd/1 -> AbsSpd/3(Vhe_Spd)
  ObsVelXAbs_s/1 -> [Fus_ObjVxAbs], AbsSpd/1(ObsVelXAbs_s)
  ObsVelYAbs_s/1 -> [Fus_ObjVyAbs], AbsSpd/2(ObsVelYAbs_s)
  [Veh_AEBSta] -> QuaCorrect/3(Veh_AEBFunState)
  Fus_Type/1 -> HeadAngle/2(Fus_Type)
  ObsAngle_s/1 -> [Fus_ObjAngle], HeadAngle/3(ObsAngle_s)
  ObsAngleSTD_s/1 -> HeadAngle/4(ObsAngleSTD_s)
  [Obj_ID] -> HeadAngle/1(Obj_ID)
  Constant/1 -> Switch/2
  [Obj_Dy] -> Switch/3
  Switch/1 -> Fus_ObjDy/1
  Constant1/1 -> Switch1/2
  Constant2/1 -> Switch2/2
  Constant3/1 -> Switch3/2
  Constant4/1 -> Switch4/2
  Switch3/1 -> Fus_ObjVy/1
  Switch4/1 -> Fus_ObjAy/1
  AccCorrect/1(Fus_ObjAy) -> Switch4/1
  [Obj_Dx] -> Switch1/3
  Switch2/1 -> Fus_ObjVx/1
  [Obj_Vx] -> Switch2/3
  [Obj_Vy] -> Switch3/3
  [Obj_AccX] -> Switch4/3
  Switch5/1 -> Fus_ObjAx/1
  Constant5/1 -> Switch5/2
  AccCorrect/2(Fus_ObjAx) -> Switch5/1
  [Obj_AccY] -> Switch5/3
  Constant12/1 -> Switch12/2
  [Fus_ObjVxAbs] -> Switch12/3
  Switch12/1 -> Fus_ObjVxAbs/1
  AbsSpd/1(Fus_ObjVxAbs) -> Switch12/1
  Switch13/1 -> Fus_ObjVyAbs/1
  AbsSpd/2(Fus_ObjVyAbs) -> Switch13/1
  [Fus_ObjVyAbs] -> Switch13/3
  Constant13/1 -> Switch13/2
  [Fus_ObjConfidence] -> Fus_ObjQuality/1
  Switch15/1 -> Fus_ObjHeadAngle/1
  HeadAngle/1(Fus_ObjHeadAngle) -> Switch15/1
  [Fus_ObjAngle] -> Switch15/3
  Constant15/1 -> Switch15/2
  [Obj_VxCr] -> HeadAngle/5(Obj_VxCr)
  [Obj_VyCr] -> HeadAngle/6(Obj_VyCr)
  [Obj_VxCr] -> AbsSpd/6(Obj_VxCr)
  [Obj_VyCr] -> AbsSpd/7(Obj_VyCr)
  [Obj_VyCr] -> AccCorrect/5(Obj_vy)
  [Obj_VxCr] -> AccCorrect/6(Obj_Vx)
  QuaCorrect/1(Fus_ObjQuality) -> Terminator/1
  [Obj_AccX] -> DxCorrect/6(Obj_AccX)
  Switch1/1 -> Fus_ObjDx/1
  [Obj_ID] -> AbsSpd/4(Obj_Id)
  [Obj_ID] -> AccCorrect/8(Obj_Id)

== system TosAeb/AEB_Objsel/TA_Processor/PreProcessor/ObjectPrecessor/TAObjInfoCalc/For Each Subsystem/Info_Check1/Dummy_Trans ==
  ObsPosXSTD_s : Inport
  ObsPosYSTD_s : Inport  Port=2
  ObsVelXSTD_s : Inport  Port=3
  ObsVelYSTD_s : Inport  Port=4
  ObsAngleSTD_s : Inport  Port=5
  ObsCenterAngle_s : Inport  Port=6
  ObsAccX_s : Inport  Port=7
  ObsAccY_s : Inport  Port=8
  Signal Conversion{,1..7} (8 alike) : SignalConversion  OverrideOpt=off
  Fus_dxSTD : Outport
  Fus_dySTD : Outport  Port=2
  Fus_VelxSTD : Outport  Port=3
  Fus_VelySTD : Outport  Port=4
  Fus_HeadAngleSTD : Outport  Port=5
  Fus_CenterAngle : Outport  Port=6
  Fus_AxAbs : Outport  Port=7
  Fus_AyAbs : Outport  Port=8
  wires:
  ObsPosXSTD_s/1 -> Signal Conversion/1
  Signal Conversion/1 -> Fus_dxSTD/1
  ObsPosYSTD_s/1 -> Signal Conversion1/1
  Signal Conversion1/1 -> Fus_dySTD/1
  ObsVelXSTD_s/1 -> Signal Conversion2/1
  Signal Conversion2/1 -> Fus_VelxSTD/1
  ObsVelYSTD_s/1 -> Signal Conversion3/1
  Signal Conversion3/1 -> Fus_VelySTD/1
  ObsAngleSTD_s/1 -> Signal Conversion4/1
  Signal Conversion4/1 -> Fus_HeadAngleSTD/1
  ObsCenterAngle_s/1 -> Signal Conversion5/1
  Signal Conversion5/1 -> Fus_CenterAngle/1
  ObsAccX_s/1 -> Signal Conversion6/1
  Signal Conversion6/1 -> Fus_AxAbs/1
  ObsAccY_s/1 -> Signal Conversion7/1
  Signal Conversion7/1 -> Fus_AyAbs/1

== system TosAeb/AEB_Objsel/TA_Processor/PreProcessor/ObjectPrecessor/TAObjInfoCalc/For Each Subsystem/Info_Check1/FusType ==
  note: 0x0: Unknow
      | 0x1: Fusion
      | 0x2: Camera
      | 0x3: Radar
  Constant : Constant  Value=2  OutDataTypeStr=uint8  SampleTime=-1
  Fus_ObjfusType : Outport
  wires:
  Constant/1 -> Fus_ObjfusType/1

== system TosAeb/AEB_Objsel/TA_Processor/PreProcessor/ObjectPrecessor/TAObjInfoCalc/For Each Subsystem/Info_Check1/ID_check ==
  note: 主要检查是否有的目标存在ID，但是有效性、存在周期及置信度不符合要求的情况
  ObsID_u8 : Inport
  ObsValid_u8 : Inport  Port=2
  ObsAge_u16 : Inport  Port=3
  ObsConfidence_u8 : Inport  Port=4
  IDValid_check : Subsystem  TreatAsAtomicUnit=on
  ID_Judge : Subsystem
  If : If  IfExpression=u1 == 1
  Invalid : Subsystem  TreatAsAtomicUnit=on
  Merge : Merge
  Merge1 : Merge
  Fus_ObjID : Outport
  ID_Change : Outport  Port=2
  wires:
  ObsID_u8/1 -> ID_Judge/1(ObsID_u8), IDValid_check/1(ObsID_u8), Invalid/1(ObsID_u8)
  ID_Judge/1(ID_Invalid) -> If/1
  If/1 -> Invalid/Action
  If/2 -> IDValid_check/Action
  ObsConfidence_u8/1 -> IDValid_check/4(ObsConfidence_u8)
  ObsAge_u16/1 -> IDValid_check/3(ObsAge_u16)
  ObsValid_u8/1 -> IDValid_check/2(ObsValid_u8)
  Invalid/1(Fus_ObjID) -> Merge/1
  IDValid_check/1(Fus_ObjID) -> Merge/2
  Merge/1 -> Fus_ObjID/1
  Invalid/2(ID_Change) -> Merge1/1
  IDValid_check/2(ID_Change) -> Merge1/2
  Merge1/1 -> ID_Change/1

== system TosAeb/AEB_Objsel/TA_Processor/PreProcessor/ObjectPrecessor/TAObjInfoCalc/For Each Subsystem/Info_Check1/LaneAssign_check ==
  ID_Change : Inport
  ObsID_u8 : Inport  Port=2
  ObsLaneAssign_u8 : Inport  Port=3
  ObsPosX_s : Inport  Port=4
  "ObsPosY_s " : Inport  Port=5
  Constant : Constant  Value=P_TA_PrmForLaneWidthThred
  LaneAssign_Cal : MATLAB Function  TreatAsAtomicUnit=on
  Fus_ObjLaneAssign : Outport
  wires:
  ObsID_u8/1 -> LaneAssign_Cal/2(ObsID_u8)
  ID_Change/1 -> LaneAssign_Cal/1(ID_Change)
  ObsLaneAssign_u8/1 -> LaneAssign_Cal/3(ObsLaneAssign_u8)
  ObsPosX_s/1 -> LaneAssign_Cal/4(ObsPosX_s)
  "ObsPosY_s "/1 -> LaneAssign_Cal/5(ObsPosY_s)
  Constant/1 -> LaneAssign_Cal/6(P_TA_PrmForLaneWidthThred)
  LaneAssign_Cal/1(Fus_ObjLaneAssign) -> Fus_ObjLaneAssign/1

== system TosAeb/AEB_Objsel/TA_Processor/PreProcessor/ObjectPrecessor/TAObjInfoCalc/For Each Subsystem/Info_Check1/Orientation_check ==
  note: ObsID_u8
      | ObsMotionCategory_u8
      | ObsPedOrientation_u8
      | ObsPosX_s
      | ObsPosY_s
      | ObsVelX_s
      | ObsVelY_s
      | ObsVelXAbs_s
      | ObsVelYAbs_s
      | ObsConerPntX_s
      | ObsCutInDis_s
      | ObsConerPntY_s
      | ObsCutInFlg_u8
      | ObsCutInSpd_s
      | ObsCutInSpdValid_b
  ID_Change : Inport
  Fus_ObsType : Inport  Port=2
  Obj_Movingstate : Inport  Port=3
  ObsID_u8 : Inport  Port=4
  ObsMotionCategory_u8 : Inport  Port=5
  ObsPedOrientation_u8 : Inport  Port=6
  ObsPosX_s : Inport  Port=7
  ObsPosY_s : Inport  Port=8
  ObsVelY_s : Inport  Port=9
  ObsVelX_s : Inport  Port=10
  ObsVelXAbs_s : Inport  Port=11
  ObsVelYAbs_s : Inport  Port=12
  ObsCutInFlg_u8 : Inport  Port=13
  ObsCutInSpd_s : Inport  Port=14
  ObsCutInSpdValid_b : Inport  Port=15
  ObsAngle_s : Inport  Port=16
  ObsPosType_u8 : Inport  Port=17
  Add : Sum
  Delay : Delay  DelayLength=50
  Math Function : Math  Operator=square  SaturateOnIntegerOverflow=on
  Math Function1 : Math  Operator=square  SaturateOnIntegerOverflow=on
  Orientation_Classify : MATLAB Function  Commented=on  TreatAsAtomicUnit=on
  Orientation_Classify1 : MATLAB Function  Commented=on  TreatAsAtomicUnit=on
  Orientation_Classify2 : MATLAB Function  Commented=on  TreatAsAtomicUnit=on
  Orientation_Classify3 : MATLAB Function  TreatAsAtomicUnit=on
  Sqrt : Sqrt  SaturateOnIntegerOverflow=on
  Terminator : Terminator
  Terminator1 : Terminator
  Terminator_1 : Terminator
  Vyaverage : Subsystem
  Fus_ObjOrientation : Outport
  wires:
  ObsCutInSpd_s/1 -> Orientation_Classify3/12(ObsCutInSpd_s)
  Math Function/1 -> Add/1
  [ObsVelYAbs_s] -> Vyaverage/1(ObsVelYAbs_s), Math Function1/1
  [ObsVelXAbs_s] -> Math Function/1
  Sqrt/1 -> Orientation_Classify3/17(ObsSpdSqrt)
  ObsPedOrientation_u8/1 -> Orientation_Classify3/5(ObsPedOrientation_u8)
  ObsCutInSpdValid_b/1 -> Orientation_Classify3/13(ObsCutInSpdValid_b)
  ObsVelXAbs_s/1 -> [ObsVelXAbs_s], Orientation_Classify3/9(ObsVelXAbs_s)
  ObsPosType_u8/1 -> Orientation_Classify3/15(ObsPosType_u8)
  ObsPosX_s/1 -> Orientation_Classify3/6(ObsPosX_s)
  [ObsPosY_s] -> Delay/1
  ID_Change/1 -> Orientation_Classify3/1(ID_Change)
  ObsMotionCategory_u8/1 -> Orientation_Classify3/4(ObsMotionCategory_u8)
  Math Function1/1 -> Add/2
  ObsVelY_s/1 -> Orientation_Classify3/8(ObsVelY_s)
  ObsVelX_s/1 -> Terminator/1
  Delay/1 -> Orientation_Classify3/16(ObsPosYDelay)
  ObsVelYAbs_s/1 -> [ObsVelYAbs_s], Orientation_Classify3/10(ObsVelYAbs_s)
  ObsAngle_s/1 -> Orientation_Classify3/14(ObsAngle_s)
  ObsPosY_s/1 -> [ObsPosY_s], Orientation_Classify3/7(ObsPosY_s)
  Add/1 -> Sqrt/1
  ObsID_u8/1 -> Orientation_Classify3/3(ObsID_u8)
  ObsCutInFlg_u8/1 -> Orientation_Classify3/11(ObsCutInFlg_u8)
  Fus_ObsType/1 -> Orientation_Classify3/2(Fus_ObsType)
  Orientation_Classify3/1(Fus_ObjOrientation) -> Fus_ObjOrientation/1
  Vyaverage/1(Obs_Vyaverage) -> Terminator1/1
  Obj_Movingstate/1 -> Terminator_1/1

== system TosAeb/AEB_Objsel/TA_Processor/PreProcessor/ObjectPrecessor/TAObjInfoCalc/For Each Subsystem/Info_Check1/State_check ==
  note: ID_Change
      | Fus_ObsType
      | ObsID_u8
      | ObsMeasureStatus_u8
      | ObsVelX_s
      | ObsVelY_s
      | ObsAccX_s
      | ObsAccY_s
      | ObsVelXAbs_s
      | ObsVelYAbs_s
  ID_Change : Inport
  Fus_ObsType : Inport  Port=2
  ObsID_u8 : Inport  Port=3
  ObsMeasureStatus_u8 : Inport  Port=4
  ObsVelXAbs_s : Inport  Port=5
  ObsVelYAbs_s : Inport  Port=6
  Spd_C : Subsystem
  State_Classify1 : MATLAB Function  TreatAsAtomicUnit=on
  State_Classify2 : MATLAB Function  Commented=on  TreatAsAtomicUnit=on
  Stop_Memory : Stateflow chart  TreatAsAtomicUnit=on
  Fus_ObjState : Outport
  wires:
  ObsVelXAbs_s/1 -> [ObsVelXAbs_s], State_Classify1/5(ObsVelXAbs_s)
  ObsVelYAbs_s/1 -> [ObsVelYAbs_s], State_Classify1/6(ObsVelYAbs_s)
  ObsMeasureStatus_u8/1 -> State_Classify1/4(ObsMeasureStatus_u8)
  ObsID_u8/1 -> [ID], State_Classify1/3(ObsID_u8)
  Fus_ObsType/1 -> State_Classify1/2(Fus_ObsType)
  ID_Change/1 -> State_Classify1/1(ID_Change), [ID_Change]
  [ID_Change] -> Stop_Memory/1(ID_Change)
  [ID] -> Stop_Memory/2(ID)
  [ObsVelYAbs_s] -> Spd_C/2(ObsVelYAbs_s)
  [ObsVelXAbs_s] -> Spd_C/1(ObsVelXAbs_s)
  Spd_C/1(Spd) -> Stop_Memory/3(Spd)
  Stop_Memory/1(Spd_Stay) -> State_Classify1/7(Spd_Stay)
  State_Classify1/1(Fus_ObjState) -> Fus_ObjState/1

== system TosAeb/AEB_Objsel/TA_Processor/PreProcessor/ObjectPrecessor/TAObjInfoCalc/For Each Subsystem/Info_Check1/Type_Precheck ==
  ID_Change : Inport
  ObsID_u8 : Inport  Port=2
  ObsMotionCategory_u8 : Inport  Port=3
  ObsMotionStatus_u8 : Inport  Port=4
  ObsClass_u8 : Inport  Port=5
  ObsVhSubType_u8 : Inport  Port=6
  ObsPedSubType_u8 : Inport  Port=7
  ObsPedPos_u8 : Inport  Port=8
  ObsLength_s : Inport  Port=9
  ObsWidth_s : Inport  Port=10
  ObsVelXAbs_s : Inport  Port=11
  ObsVelYAbs_s : Inport  Port=12
  Constant : Constant  Value=P_TA_PrmForMotorSpdThred
  ObsSpd_Calculate : Subsystem
  Terminator{,1..3} (4 alike) : Terminator
  Type_Classify1 : MATLAB Function  Commented=on  TreatAsAtomicUnit=on
  Type_comfig : MATLAB Function  TreatAsAtomicUnit=on
  Fus_ObType : Outport
  wires:
  ObsSpd_Calculate/1(ObsSpd_s) -> Type_comfig/7(ObsSpd_s)  "ObsSpd_Calculate"
  ObsVelXAbs_s/1 -> ObsSpd_Calculate/1(ObsVelXAbs_s)
  ObsPedPos_u8/1 -> Type_comfig/6(ObsPedPos_u8)  "ObsPedPos_u8"
  ObsPedSubType_u8/1 -> Type_comfig/5(ObsPedSubType_u8)  "ObsPedSubType_u8"
  ObsVelYAbs_s/1 -> ObsSpd_Calculate/2(ObsVelYAbs_s)
  ObsVhSubType_u8/1 -> Type_comfig/4(ObsVhSubType_u8)  "ObsVhSubType_u8"
  ID_Change/1 -> Type_comfig/1(ID_Change)  "ID_Change"
  ObsClass_u8/1 -> Type_comfig/3(ObsClass_u8)  "ObsClass_u8"
  ObsID_u8/1 -> Type_comfig/2(ObsID_u8)  "ObsID_u8"
  Constant/1 -> Type_comfig/8(P_TA_PrmForMotorSpdThred)
  Type_comfig/1(Fus_ObjType) -> Fus_ObType/1
  ObsMotionCategory_u8/1 -> Terminator/1  "ObsMotionCategory_u8"
  ObsMotionStatus_u8/1 -> Terminator1/1  "ObsMotionStatus_u8"
  ObsLength_s/1 -> Terminator2/1  "ObsLength_s"
  ObsWidth_s/1 -> Terminator3/1  "ObsWidth_s"

== system TosAeb/AEB_Objsel/TA_Processor/PreProcessor/ObjectPrecessor/TAObjInfoCalc/For Each Subsystem/Info_Check1/Type_Process ==
  Obj_ID : Inport
  Obj_Length : Inport  Port=2
  Obj_Width : Inport  Port=3
  Obj_Height : Inport  Port=4
  Obj_dx : Inport  Port=5
  Obj_type : Inport  Port=6
  abs_Spd : Inport  Port=7
  Constant : Constant  Value=P_TA_ObjTruckLengthPara
  Constant1 : Constant  Commented=on  Value=P_TA_ObjTruckWidthPara
  Constant10 : Constant  Commented=on  Value=P_TA_ObjBusLengthPara
  Constant11 : Constant  Commented=on  Value=P_TA_ObjBusWidthPara
  Constant12 : Constant  Commented=on  Value=P_TA_ObjLongthValidFactor
  Constant13 : Constant  Commented=on  Value=P_TA_ObjWidthValidFactor
  Constant14 : Constant  Value=P_TA_ObjMotorVehSpdThd
  Constant15 : Constant  Value=P_TA_ObjPedestrainSpdThd
  Constant2 : Constant  Commented=on  Value=P_TA_ObjCarLengthPara
  Constant3 : Constant  Commented=on  Value=P_TA_ObjCarWidthPara
  Constant4 : Constant  Commented=on  Value=P_TA_ObjMotorBicycleLengthPara
  Constant5 : Constant  Commented=on  Value=P_TA_ObjMotorBicycleWidthPara
  Constant6 : Constant  Commented=on  Value=P_TA_ObjBicycleLengthPara
  Constant7 : Constant  Commented=on  Value=P_TA_ObjBicycleWidthPara
  Constant8 : Constant  Commented=on  Value=P_TA_ObjPedestrainLengthPara
  Constant9 : Constant  Commented=on  Value=P_TA_ObjPedestrainWidthPara
  From : From  Commented=on  GotoTag=TA_ObjInfo
  From1 : From  Commented=on  GotoTag=VehSelfData
  Heading : Subsystem  Commented=on
  MATLAB Function2 : MATLAB Function  Commented=on  TreatAsAtomicUnit=on
  ObjType_rebuild : MATLAB Function  TreatAsAtomicUnit=on
  Terminator1 : Terminator
  Fus_ObjType : Outport
  Fus_ObjLength : Outport  Port=2
  Fus_ObjWidth : Outport  Port=3
  Fus_ObjHight : Outport  Port=4
  wires:
  From/1 -> Heading/2(TA_ObjInfo)
  Obj_type/1 -> ObjType_rebuild/2(Obj_type)
  From1/1 -> Heading/3(VehSelfData)
  Obj_Width/1 -> ObjType_rebuild/4(Obj_Width)
  ObjType_rebuild/4(C_ObjHight) -> Fus_ObjHight/1
  Obj_Length/1 -> ObjType_rebuild/3(Obj_Length)
  Obj_ID/1 -> ObjType_rebuild/1(Obj_ID)
  Obj_dx/1 -> ObjType_rebuild/6(Obj_dx)
  Obj_Height/1 -> ObjType_rebuild/5(Obj_Height)
  abs_Spd/1 -> ObjType_rebuild/7(abs_Spd)
  Constant14/1 -> ObjType_rebuild/9(P_TA_ObjMotorVehSpdThd)
  Constant/1 -> ObjType_rebuild/8(P_TA_ObjTruckLengthPara)
  Constant15/1 -> ObjType_rebuild/10(P_TA_ObjPedestrainSpdThd)
  ObjType_rebuild/1(C_ObjType) -> Fus_ObjType/1
  ObjType_rebuild/2(C_ObjLength) -> Fus_ObjLength/1
  ObjType_rebuild/3(C_ObjWidth) -> Fus_ObjWidth/1
  ObjType_rebuild/5(C_Objdx) -> Terminator1/1

== system TosAeb/AEB_Objsel/TA_Processor/PreProcessor/VehiclePreProcessor/CalculateCurvature/LowPassFilter/LP1_1/S16_Lag_Filter ==
  unfiltered_input : Inport
  filtered_value_n_1 : Inport  Port=2
  filter_gain : Inport  Port=3
  Product : Product  Inputs=**  InputSameDT=on  OutDataTypeStr=Inherit: Same as first input
      settings: AttributesFormatString=InputSameDT=%<InputSameDT>
  Product1 : Product  Inputs=**  InputSameDT=on  OutDataTypeStr=Inherit: Same as first input
      settings: AttributesFormatString=InputSameDT=%<InputSameDT>
  Sum1 : Sum  Inputs=-+  InputSameDT=on  OutDataTypeStr=Inherit: Same as first input
      settings: AttributesFormatString=InputSameDT=%<InputSameDT>
  Sum2 : Sum  InputSameDT=on  OutDataTypeStr=Inherit: Same as first input
      settings: AttributesFormatString=InputSameDT=%<InputSameDT>
  filtered_output : Outport
  wires:
  Sum2/1 -> filtered_output/1  "filtered_output"
  Product1/1 -> Sum2/1
  Sum1/1 -> Sum2/2
  Product/1 -> Sum1/1
  filtered_value_n_1/1 -> Product/2, Sum1/2
  filter_gain/1 -> Product1/2, Product/1
  unfiltered_input/1 -> Product1/1

== system TosAeb/AEB_Objsel/TA_Processor/PreProcessor/VehiclePreProcessor/CalculateCurvature/StaticBicycleModelC0/CalculateCurvatureCoG/RadiusCoGJudge ==
  Con : Inport
  CurvatureCoG : Inport  Port=2
  Compare To Constant : simulink/Logic and Bit Operations/Compare To Constant  relop=>=  const=100000  OutDataTypeStr=boolean
  Compare To Constant1 : simulink/Logic and Bit Operations/Compare To Constant  relop=<  const=1e-6  OutDataTypeStr=boolean
  Constant : Constant  Value=1e-6  OutDataTypeStr=single
  Constant4 : Constant  Value=100000  OutDataTypeStr=single
  Divide : Product  Inputs=*/
  Switch : Switch  Criteria=u2 ~= 0
  Switch1 : Switch  Criteria=u2 > Threshold
  RadiusCoG : Outport
  wires:
  Divide/1 -> Switch/3, Compare To Constant/1  "RadiusCoG"
  Constant4/1 -> Switch/1
  Compare To Constant/1 -> Switch/2
  CurvatureCoG/1 -> Switch1/3, Compare To Constant1/1
  Switch/1 -> RadiusCoG/1
  Con/1 -> Divide/1
  Compare To Constant1/1 -> Switch1/2
  Constant/1 -> Switch1/1
  Switch1/1 -> Divide/2

== system TosAeb/AEB_Objsel/TA_Processor/PreProcessor/VehiclePreProcessor/CalculateCurvature/StaticBicycleModelC0/CalculateDistanceToAxles/Rename ==
  note: Block intended for fooling the model advisor, which want the signal names to
      | match the port names and which only allows signal renaming in combination
      | with transformative operations. The block has no effect on the signal and is
      | intended to replace, e.g., gain blocks with one as its multiplication factor.
      | See MAAB jm_0010: Port block names in Simulink models.
  Name_in : Inport
      settings: AttributesFormatString=DT=%<OutDataTypeStr>
  Name_out : Outport
      settings: AttributesFormatString=DT=%<OutDataTypeStr>
  wires:
  Name_in/1 -> Name_out/1  "Name"

== system TosAeb/AEB_Objsel/TA_Processor/PreProcessor/VehiclePreProcessor/CalculateCurvature/StaticBicycleModelC0/CalculateWheelAngle/SafeDivide ==
  Numerator : Inport  OutDataTypeStr=DataType
      settings: AttributesFormatString=DT=%<OutDataTypeStr>
  Denominator : Inport  Port=2  OutDataTypeStr=DataType
      settings: AttributesFormatString=DT=%<OutDataTypeStr>
  SafeDivide : MATLAB Function
  Quotient : Outport  OutDataTypeStr=DataType
      settings: AttributesFormatString=DT=%<OutDataTypeStr>
  wires:
  SafeDivide/1(Quotient) -> Quotient/1
  Denominator/1 -> SafeDivide/2(Denominator)  "Denominator"
  Numerator/1 -> SafeDivide/1(Numerator)  "Numerator"

== system TosAeb/AEB_Objsel/TA_Processor/PreProcessor/VehiclePreProcessor/CalculateCurvature/StaticBicycleModelC0/IntermediateCalculation/SafeDivide ==
  Numerator : Inport  OutDataTypeStr=DataType
      settings: AttributesFormatString=DT=%<OutDataTypeStr>
  Denominator : Inport  Port=2  OutDataTypeStr=DataType
      settings: AttributesFormatString=DT=%<OutDataTypeStr>
  SafeDivide : MATLAB Function
  Quotient : Outport  OutDataTypeStr=DataType
      settings: AttributesFormatString=DT=%<OutDataTypeStr>
  wires:
  SafeDivide/1(Quotient) -> Quotient/1
  Denominator/1 -> SafeDivide/2(Denominator)  "Denominator"
  Numerator/1 -> SafeDivide/1(Numerator)  "Numerator"

== system TosAeb/AEB_Objsel/TA_Processor/PreProcessor/VehiclePreProcessor/CalculateCurvature/StaticBicycleModelC1/CalculateDistanceToAxles/Rename ==
  note: Block intended for fooling the model advisor, which want the signal names to
      | match the port names and which only allows signal renaming in combination
      | with transformative operations. The block has no effect on the signal and is
      | intended to replace, e.g., gain blocks with one as its multiplication factor.
      | See MAAB jm_0010: Port block names in Simulink models.
  Name_in : Inport
      settings: AttributesFormatString=DT=%<OutDataTypeStr>
  Name_out : Outport
      settings: AttributesFormatString=DT=%<OutDataTypeStr>
  wires:
  Name_in/1 -> Name_out/1  "Name"

== system TosAeb/AEB_Objsel/TA_Processor/PreProcessor/VehiclePreProcessor/CalculateCurvature/StaticBicycleModelC1/CalculateWheelAngle/SafeDivide ==
  Numerator : Inport  OutDataTypeStr=DataType
      settings: AttributesFormatString=DT=%<OutDataTypeStr>
  Denominator : Inport  Port=2  OutDataTypeStr=DataType
      settings: AttributesFormatString=DT=%<OutDataTypeStr>
  SafeDivide : MATLAB Function
  Quotient : Outport  OutDataTypeStr=DataType
      settings: AttributesFormatString=DT=%<OutDataTypeStr>
  wires:
  SafeDivide/1(Quotient) -> Quotient/1
  Denominator/1 -> SafeDivide/2(Denominator)  "Denominator"
  Numerator/1 -> SafeDivide/1(Numerator)  "Numerator"

== system TosAeb/AEB_Objsel/TA_Processor/PreProcessor/VehiclePreProcessor/CalculateCurvature/StaticBicycleModelC1/IntermediateCalculation/SafeDivide ==
  Numerator : Inport  OutDataTypeStr=DataType
      settings: AttributesFormatString=DT=%<OutDataTypeStr>
  Denominator : Inport  Port=2  OutDataTypeStr=DataType
      settings: AttributesFormatString=DT=%<OutDataTypeStr>
  SafeDivide : MATLAB Function
  Quotient : Outport  OutDataTypeStr=DataType
      settings: AttributesFormatString=DT=%<OutDataTypeStr>
  wires:
  SafeDivide/1(Quotient) -> Quotient/1
  Denominator/1 -> SafeDivide/2(Denominator)  "Denominator"
  Numerator/1 -> SafeDivide/1(Numerator)  "Numerator"

== system TosAeb/AEB_Objsel/TA_Processor/PreProcessor/VehiclePreProcessor/PathEstimn_Calculate/For Iterator PahEstimn/ComputeVehicleStateAtT1/AccelerationAtT1 ==
  AT0 : Inport
  StopsWithinSegment : Inport  Port=2
  Constant : Constant  Value=single(0)
  Switch : Switch  Criteria=u2 ~= 0
  A : Outport
  wires:
  StopsWithinSegment/1 -> Switch/2
  AT0/1 -> Switch/3
  Constant/1 -> Switch/1
  Switch/1 -> A/1  "A"

== system TosAeb/AEB_Objsel/TA_Processor/PreProcessor/VehiclePreProcessor/PathEstimn_Calculate/For Iterator PahEstimn/ComputeVehicleStateAtT1/ComputeHeadingAngle ==
  VLgt : Inport
  VLat : Inport  Port=2
  HeadingAngleT0 : Inport  Port=3
  Abs : Abs
  Atan2 : Trigonometry  Operator=atan2
  Compare To Zero : simulink/Logic and Bit Operations/Compare To Zero  relop=>  OutDataTypeStr=boolean
  Switch : Switch  Criteria=u2 ~= 0
  HeadingAngle : Outport
  wires:
  Atan2/1 -> Switch/1, Abs/1  "HeadingFromVelocity"
  Abs/1 -> Compare To Zero/1
  Compare To Zero/1 -> Switch/2
  HeadingAngleT0/1 -> Switch/3
  Switch/1 -> HeadingAngle/1
  VLgt/1 -> Atan2/2
  VLat/1 -> Atan2/1
